simulink model "test03"  (R2022a)
settings: Solver=FixedStepAuto  StartTime=0.0  StopTime=0.05  FixedStep=1e-4  RelTol=1e-3
values from neighbouring files (symbols this model uses):
  A = 0.01    [parameter_load3.m]
  d = 2.6    [parameter_load3.m]
  I = 3055e-8    (= 3.055e-05)    [parameter_load3.m]
  jv = 1064400    [parameter_load3.m]
  kf = 6.67e7    (= 66700000)    [parameter_load3.m]
  kv = 400000    [parameter_load3.m]
  L = 210    [parameter_load3.m]
  m = 7800*(7684e-6)    (= 59.9352)    [parameter_load3.m]
  mv = 42400    [parameter_load3.m]
  position = 0.5*L    (= 105)    [parameter_load3.m]
  r = 840/1000/2    (= 0.42)    [parameter_load3.m]
  v = 100/3.6    (= 27.7777777778)    [parameter_load3.m]
(part 49 of 73)

== system test03/Subsystem8/Subsystem/Subsystem/Subsystem5/Subsystem2 ==
  An1 : Inport
  theta_n1 : Inport  Port=2
  theta_n2 : Inport  Port=3
  theta_n3 : Inport  Port=4
  Sn : Inport  Port=5
  static_n : Inport  Port=6
  Omiga_bn : Inport  Port=7
  Omiga_n : Inport  Port=8
  Add : Sum  Inputs=-+
  Add1 : Sum  Inputs=+-+-
  Add{2..4,6} (4 alike) : Sum
  Add5 : Sum  Inputs=+-
  Clock : Clock
  Clock1 : Clock
  Clock2 : Clock
  Constant : Constant
  Cos : Trigonometry  Operator=cos
  Cos1 : Trigonometry  Operator=cos
  Cos2 : Trigonometry  Operator=cos
  Divide : Product  Inputs=/*
  Gain : Gain  Gain=2
  Gain1 : Gain  Gain=2
  Product{,1..5} (6 alike) : Product
  Square : Math  Operator=square
  Out1 : Outport
  wires:
  Divide/1 -> Product5/1
  Add1/1 -> Product5/2
  Product5/1 -> Out1/1
  Product4/1 -> Add1/2
  Cos2/1 -> Product4/1
  Add6/1 -> Cos2/1
  Product3/1 -> Add6/1
  Clock2/1 -> Product3/2
  Add5/1 -> Product3/1
  theta_n2/1 -> Add6/2
  Product2/1 -> Add1/3
  Cos1/1 -> Product2/2
  Add4/1 -> Cos1/1
  theta_n3/1 -> Add4/2
  Product1/1 -> Add4/1
  Add3/1 -> Product1/1
  Clock1/1 -> Product1/2
  Omiga_bn/1 -> Add3/1, Add5/1
  Cos/1 -> Add1/4
  Add2/1 -> Cos/1
  theta_n1/1 -> Add2/2
  Product/1 -> Add2/1
  Clock/1 -> Product/2
  Gain1/1 -> Product/1
  Omiga_n/1 -> Gain1/1, Add3/2, Add5/2
  An1/1 -> Add1/1
  Gain/1 -> Divide/1
  Add/1 -> Gain/1
  Constant/1 -> Add/2
  Square/1 -> Add/1
  Sn/1 -> Product2/1, Product4/2, Square/1
  static_n/1 -> Divide/2

== system test03/Subsystem8/Subsystem/Subsystem/Subsystem5/Subsystem3 ==
  An1 : Inport
  theta_n1 : Inport  Port=2
  theta_n2 : Inport  Port=3
  theta_n3 : Inport  Port=4
  Sn : Inport  Port=5
  static_n : Inport  Port=6
  Omiga_bn : Inport  Port=7
  Omiga_n : Inport  Port=8
  Add : Sum  Inputs=-+
  Add1 : Sum  Inputs=+-+-
  Add{2..4,6} (4 alike) : Sum
  Add5 : Sum  Inputs=+-
  Clock : Clock
  Clock1 : Clock
  Clock2 : Clock
  Constant : Constant
  Cos : Trigonometry  Operator=cos
  Cos1 : Trigonometry  Operator=cos
  Cos2 : Trigonometry  Operator=cos
  Divide : Product  Inputs=/*
  Gain : Gain  Gain=2
  Gain1 : Gain  Gain=2
  Product{,1..5} (6 alike) : Product
  Square : Math  Operator=square
  Out1 : Outport
  wires:
  static_n/1 -> Divide/2
  Sn/1 -> Square/1, Product4/2, Product2/1
  Square/1 -> Add/1
  Constant/1 -> Add/2
  Add/1 -> Gain/1
  Gain/1 -> Divide/1
  An1/1 -> Add1/1
  Omiga_n/1 -> Add5/2, Add3/2, Gain1/1
  Gain1/1 -> Product/1
  Clock/1 -> Product/2
  Product/1 -> Add2/1
  theta_n1/1 -> Add2/2
  Add2/1 -> Cos/1
  Cos/1 -> Add1/4
  Omiga_bn/1 -> Add5/1, Add3/1
  Clock1/1 -> Product1/2
  Add3/1 -> Product1/1
  Product1/1 -> Add4/1
  theta_n3/1 -> Add4/2
  Add4/1 -> Cos1/1
  Cos1/1 -> Product2/2
  Product2/1 -> Add1/3
  theta_n2/1 -> Add6/2
  Add5/1 -> Product3/1
  Clock2/1 -> Product3/2
  Product3/1 -> Add6/1
  Add6/1 -> Cos2/1
  Cos2/1 -> Product4/1
  Product4/1 -> Add1/2
  Product5/1 -> Out1/1
  Add1/1 -> Product5/2
  Divide/1 -> Product5/1

== system test03/Subsystem8/Subsystem/Subsystem/Subsystem6/Subsystem ==
  imod : Inport
  r : Inport  Port=2
  k : Inport  Port=3
  F : Inport  Port=4
  Add : Sum  Inputs=+-
  Add1 : Sum
  Add2 : Sum
  Add3 : Sum  Inputs=-+
  Add4 : Sum  Inputs=-+
  Add5 : Sum  Inputs=--
  Constant : Constant
  Constant1 : Constant  Value=0
  Constant2 : Constant  Value=4
  Constant3 : Constant  Value=kf/m
  Cos : Trigonometry  Operator=cos
  Divide : Product  Inputs=*/
  Divide1 : Product  Inputs=*/
  Gain : Gain  Gain=d
  Gain1 : Gain  Gain=pi/L
  Gain2 : Gain  Gain=E*I/m
  Gain3 : Gain  Gain=v*pi/L
  Gain4 : Gain  Gain=-2/(m*L)
  Gain5 : Gain  Gain=-1
  Gain6 : Gain  Gain=1/v
  Power : Math  Operator=pow
  Product{1..6} (6 alike) : Product
  Sqrt : Sqrt
  Square1 : Math  Operator=square
  Switch : Switch  Criteria=u2 > Threshold  Threshold=1  SaturateOnIntegerOverflow=off  InputSameDT=off
  An1 : Outport
  theta_n1 : Outport  Port=2
  theta_n2 : Outport  Port=3
  theta_n3 : Outport  Port=4
  Sn : Outport  Port=5
  static_n : Outport  Port=6
  Omiga_bn : Outport  Port=7
  Omiga_n : Outport  Port=8
  wires:
  k/1 -> Add/1
  Constant/1 -> Add/2
  Add/1 -> Gain/1
  Gain/1 -> Gain6/1
  r/1 -> Switch/2
  Constant1/1 -> Switch/3
  [tk] -> Switch/1
  Switch/1 -> [tr]
  Product4/1 -> Cos/1
  [tr] -> Add4/1
  Sqrt/1 -> Omiga_bn/1, [Omiga_bn]
  Constant2/1 -> Power/2
  Gain2/1 -> Add1/1
  Add4/1 -> Product4/2
  Add1/1 -> Sqrt/1
  Gain4/1 -> Divide1/1
  F/1 -> Gain4/1
  [imod] -> Gain1/1
  Divide/1 -> Sn/1
  Constant3/1 -> Add1/2
  Divide1/1 -> static_n/1
  Gain1/1 -> Power/1
  Gain3/1 -> Omiga_n/1, [Omiga_n]
  Power/1 -> Gain2/1
  [Omiga_bn] -> Square1/1
  Square1/1 -> Divide1/2
  [tk] -> Add4/2
  [Omiga_n] -> Divide/1
  [Omiga_n] -> Product4/1
  [Omiga_bn] -> Divide/2
  [imod] -> Gain3/1
  imod/1 -> [imod]
  Cos/1 -> An1/1
  [Omiga_n] -> Product1/1
  [tk] -> Add2/2
  Add2/1 -> Product1/2
  [tr] -> Add2/1
  Product1/1 -> Gain5/1
  Gain5/1 -> theta_n1/1
  [Omiga_bn] -> Product2/1
  [tk] -> Product2/2
  Product2/1 -> Add3/1
  Add3/1 -> theta_n2/1
  Product3/1 -> Add3/2
  [tr] -> Product3/2
  [Omiga_n] -> Product3/1
  [Omiga_n] -> Product6/1
  [tr] -> Product6/2
  Product6/1 -> Add5/2
  Add5/1 -> theta_n3/1
  Product5/1 -> Add5/1
  [tk] -> Product5/2
  [Omiga_bn] -> Product5/1
  Gain6/1 -> [tk]

== system test03/Subsystem8/Subsystem/Subsystem/Subsystem6/Subsystem1 ==
  imod : Inport
  r : Inport  Port=2
  k : Inport  Port=3
  F : Inport  Port=4
  Add : Sum  Inputs=+-
  Add1 : Sum
  Add2 : Sum
  Add3 : Sum  Inputs=-+
  Add4 : Sum  Inputs=-+
  Add5 : Sum  Inputs=--
  Constant : Constant
  Constant1 : Constant  Value=0
  Constant2 : Constant  Value=4
  Constant3 : Constant  Value=kf/m
  Cos : Trigonometry  Operator=cos
  Divide : Product  Inputs=*/
  Divide1 : Product  Inputs=*/
  Gain : Gain  Gain=d
  Gain1 : Gain  Gain=pi/L
  Gain2 : Gain  Gain=E*I/m
  Gain3 : Gain  Gain=v*pi/L
  Gain4 : Gain  Gain=-2/(m*L)
  Gain5 : Gain  Gain=-1
  Gain6 : Gain  Gain=1/v
  Power : Math  Operator=pow
  Product{1..6} (6 alike) : Product
  Sqrt : Sqrt
  Square1 : Math  Operator=square
  Switch : Switch  Criteria=u2 > Threshold  Threshold=1  SaturateOnIntegerOverflow=off  InputSameDT=off
  An1 : Outport
  theta_n1 : Outport  Port=2
  theta_n2 : Outport  Port=3
  theta_n3 : Outport  Port=4
  Sn : Outport  Port=5
  static_n : Outport  Port=6
  Omiga_bn : Outport  Port=7
  Omiga_n : Outport  Port=8
  wires:
  Gain6/1 -> [tk]
  [Omiga_bn] -> Product5/1
  [tk] -> Product5/2
  Product5/1 -> Add5/1
  Add5/1 -> theta_n3/1
  Product6/1 -> Add5/2
  [tr] -> Product6/2
  [Omiga_n] -> Product6/1
  [Omiga_n] -> Product3/1
  [tr] -> Product3/2
  Product3/1 -> Add3/2
  Add3/1 -> theta_n2/1
  Product2/1 -> Add3/1
  [tk] -> Product2/2
  [Omiga_bn] -> Product2/1
  Gain5/1 -> theta_n1/1
  Product1/1 -> Gain5/1
  [tr] -> Add2/1
  Add2/1 -> Product1/2
  [tk] -> Add2/2
  [Omiga_n] -> Product1/1
  Cos/1 -> An1/1
  imod/1 -> [imod]
  [imod] -> Gain3/1
  [Omiga_bn] -> Divide/2
  [Omiga_n] -> Product4/1
  [Omiga_n] -> Divide/1
  [tk] -> Add4/2
  Square1/1 -> Divide1/2
  [Omiga_bn] -> Square1/1
  Power/1 -> Gain2/1
  Gain3/1 -> [Omiga_n], Omiga_n/1
  Gain1/1 -> Power/1
  Divide1/1 -> static_n/1
  Constant3/1 -> Add1/2
  Divide/1 -> Sn/1
  [imod] -> Gain1/1
  F/1 -> Gain4/1
  Gain4/1 -> Divide1/1
  Add1/1 -> Sqrt/1
  Add4/1 -> Product4/2
  Gain2/1 -> Add1/1
  Constant2/1 -> Power/2
  Sqrt/1 -> [Omiga_bn], Omiga_bn/1
  [tr] -> Add4/1
  Product4/1 -> Cos/1
  Switch/1 -> [tr]
  [tk] -> Switch/1
  Constant1/1 -> Switch/3
  r/1 -> Switch/2
  Gain/1 -> Gain6/1
  Add/1 -> Gain/1
  Constant/1 -> Add/2
  k/1 -> Add/1

== system test03/Subsystem8/Subsystem/Subsystem/Subsystem6/Subsystem2 ==
  An1 : Inport
  theta_n1 : Inport  Port=2
  theta_n2 : Inport  Port=3
  theta_n3 : Inport  Port=4
  Sn : Inport  Port=5
  static_n : Inport  Port=6
  Omiga_bn : Inport  Port=7
  Omiga_n : Inport  Port=8
  Add : Sum  Inputs=-+
  Add1 : Sum  Inputs=+-+-
  Add{2..4,6} (4 alike) : Sum
  Add5 : Sum  Inputs=+-
  Clock : Clock
  Clock1 : Clock
  Clock2 : Clock
  Constant : Constant
  Cos : Trigonometry  Operator=cos
  Cos1 : Trigonometry  Operator=cos
  Cos2 : Trigonometry  Operator=cos
  Divide : Product  Inputs=/*
  Gain : Gain  Gain=2
  Gain1 : Gain  Gain=2
  Product{,1..5} (6 alike) : Product
  Square : Math  Operator=square
  Out1 : Outport
  wires:
  static_n/1 -> Divide/2
  Sn/1 -> Square/1, Product4/2, Product2/1
  Square/1 -> Add/1
  Constant/1 -> Add/2
  Add/1 -> Gain/1
  Gain/1 -> Divide/1
  An1/1 -> Add1/1
  Omiga_n/1 -> Add5/2, Add3/2, Gain1/1
  Gain1/1 -> Product/1
  Clock/1 -> Product/2
  Product/1 -> Add2/1
  theta_n1/1 -> Add2/2
  Add2/1 -> Cos/1
  Cos/1 -> Add1/4
  Omiga_bn/1 -> Add5/1, Add3/1
  Clock1/1 -> Product1/2
  Add3/1 -> Product1/1
  Product1/1 -> Add4/1
  theta_n3/1 -> Add4/2
  Add4/1 -> Cos1/1
  Cos1/1 -> Product2/2
  Product2/1 -> Add1/3
  theta_n2/1 -> Add6/2
  Add5/1 -> Product3/1
  Clock2/1 -> Product3/2
  Product3/1 -> Add6/1
  Add6/1 -> Cos2/1
  Cos2/1 -> Product4/1
  Product4/1 -> Add1/2
  Product5/1 -> Out1/1
  Add1/1 -> Product5/2
  Divide/1 -> Product5/1

== system test03/Subsystem8/Subsystem/Subsystem/Subsystem6/Subsystem3 ==
  An1 : Inport
  theta_n1 : Inport  Port=2
  theta_n2 : Inport  Port=3
  theta_n3 : Inport  Port=4
  Sn : Inport  Port=5
  static_n : Inport  Port=6
  Omiga_bn : Inport  Port=7
  Omiga_n : Inport  Port=8
  Add : Sum  Inputs=-+
  Add1 : Sum  Inputs=+-+-
  Add{2..4,6} (4 alike) : Sum
  Add5 : Sum  Inputs=+-
  Clock : Clock
  Clock1 : Clock
  Clock2 : Clock
  Constant : Constant
  Cos : Trigonometry  Operator=cos
  Cos1 : Trigonometry  Operator=cos
  Cos2 : Trigonometry  Operator=cos
  Divide : Product  Inputs=/*
  Gain : Gain  Gain=2
  Gain1 : Gain  Gain=2
  Product{,1..5} (6 alike) : Product
  Square : Math  Operator=square
  Out1 : Outport
  wires:
  Divide/1 -> Product5/1
  Add1/1 -> Product5/2
  Product5/1 -> Out1/1
  Product4/1 -> Add1/2
  Cos2/1 -> Product4/1
  Add6/1 -> Cos2/1
  Product3/1 -> Add6/1
  Clock2/1 -> Product3/2
  Add5/1 -> Product3/1
  theta_n2/1 -> Add6/2
  Product2/1 -> Add1/3
  Cos1/1 -> Product2/2
  Add4/1 -> Cos1/1
  theta_n3/1 -> Add4/2
  Product1/1 -> Add4/1
  Add3/1 -> Product1/1
  Clock1/1 -> Product1/2
  Omiga_bn/1 -> Add3/1, Add5/1
  Cos/1 -> Add1/4
  Add2/1 -> Cos/1
  theta_n1/1 -> Add2/2
  Product/1 -> Add2/1
  Clock/1 -> Product/2
  Gain1/1 -> Product/1
  Omiga_n/1 -> Gain1/1, Add3/2, Add5/2
  An1/1 -> Add1/1
  Gain/1 -> Divide/1
  Add/1 -> Gain/1
  Constant/1 -> Add/2
  Square/1 -> Add/1
  Sn/1 -> Product2/1, Product4/2, Square/1
  static_n/1 -> Divide/2

== system test03/Subsystem8/Subsystem/Subsystem/Subsystem7/Subsystem ==
  imod : Inport
  r : Inport  Port=2
  k : Inport  Port=3
  F : Inport  Port=4
  Add : Sum  Inputs=+-
  Add1 : Sum
  Add2 : Sum
  Add3 : Sum  Inputs=-+
  Add4 : Sum  Inputs=-+
  Add5 : Sum  Inputs=--
  Constant : Constant
  Constant1 : Constant  Value=0
  Constant2 : Constant  Value=4
  Constant3 : Constant  Value=kf/m
  Cos : Trigonometry  Operator=cos
  Divide : Product  Inputs=*/
  Divide1 : Product  Inputs=*/
  Gain : Gain  Gain=d
  Gain1 : Gain  Gain=pi/L
  Gain2 : Gain  Gain=E*I/m
  Gain3 : Gain  Gain=v*pi/L
  Gain4 : Gain  Gain=-2/(m*L)
  Gain5 : Gain  Gain=-1
  Gain6 : Gain  Gain=1/v
  Power : Math  Operator=pow
  Product{1..6} (6 alike) : Product
  Sqrt : Sqrt
  Square1 : Math  Operator=square
  Switch : Switch  Criteria=u2 > Threshold  Threshold=1  SaturateOnIntegerOverflow=off  InputSameDT=off
  An1 : Outport
  theta_n1 : Outport  Port=2
  theta_n2 : Outport  Port=3
  theta_n3 : Outport  Port=4
  Sn : Outport  Port=5
  static_n : Outport  Port=6
  Omiga_bn : Outport  Port=7
  Omiga_n : Outport  Port=8
  wires:
  k/1 -> Add/1
  Constant/1 -> Add/2
  Add/1 -> Gain/1
  Gain/1 -> Gain6/1
  r/1 -> Switch/2
  Constant1/1 -> Switch/3
  [tk] -> Switch/1
  Switch/1 -> [tr]
  Product4/1 -> Cos/1
  [tr] -> Add4/1
  Sqrt/1 -> Omiga_bn/1, [Omiga_bn]
  Constant2/1 -> Power/2
  Gain2/1 -> Add1/1
  Add4/1 -> Product4/2
  Add1/1 -> Sqrt/1
  Gain4/1 -> Divide1/1
  F/1 -> Gain4/1
  [imod] -> Gain1/1
  Divide/1 -> Sn/1
  Constant3/1 -> Add1/2
  Divide1/1 -> static_n/1
  Gain1/1 -> Power/1
  Gain3/1 -> Omiga_n/1, [Omiga_n]
  Power/1 -> Gain2/1
  [Omiga_bn] -> Square1/1
  Square1/1 -> Divide1/2
  [tk] -> Add4/2
  [Omiga_n] -> Divide/1
  [Omiga_n] -> Product4/1
  [Omiga_bn] -> Divide/2
  [imod] -> Gain3/1
  imod/1 -> [imod]
  Cos/1 -> An1/1
  [Omiga_n] -> Product1/1
  [tk] -> Add2/2
  Add2/1 -> Product1/2
  [tr] -> Add2/1
  Product1/1 -> Gain5/1
  Gain5/1 -> theta_n1/1
  [Omiga_bn] -> Product2/1
  [tk] -> Product2/2
  Product2/1 -> Add3/1
  Add3/1 -> theta_n2/1
  Product3/1 -> Add3/2
  [tr] -> Product3/2
  [Omiga_n] -> Product3/1
  [Omiga_n] -> Product6/1
  [tr] -> Product6/2
  Product6/1 -> Add5/2
  Add5/1 -> theta_n3/1
  Product5/1 -> Add5/1
  [tk] -> Product5/2
  [Omiga_bn] -> Product5/1
  Gain6/1 -> [tk]

== system test03/Subsystem8/Subsystem/Subsystem/Subsystem7/Subsystem1 ==
  imod : Inport
  r : Inport  Port=2
  k : Inport  Port=3
  F : Inport  Port=4
  Add : Sum  Inputs=+-
  Add1 : Sum
  Add2 : Sum
  Add3 : Sum  Inputs=-+
  Add4 : Sum  Inputs=-+
  Add5 : Sum  Inputs=--
  Constant : Constant
  Constant1 : Constant  Value=0
  Constant2 : Constant  Value=4
  Constant3 : Constant  Value=kf/m
  Cos : Trigonometry  Operator=cos
  Divide : Product  Inputs=*/
  Divide1 : Product  Inputs=*/
  Gain : Gain  Gain=d
  Gain1 : Gain  Gain=pi/L
  Gain2 : Gain  Gain=E*I/m
  Gain3 : Gain  Gain=v*pi/L
  Gain4 : Gain  Gain=-2/(m*L)
  Gain5 : Gain  Gain=-1
  Gain6 : Gain  Gain=1/v
  Power : Math  Operator=pow
  Product{1..6} (6 alike) : Product
  Sqrt : Sqrt
  Square1 : Math  Operator=square
  Switch : Switch  Criteria=u2 > Threshold  Threshold=1  SaturateOnIntegerOverflow=off  InputSameDT=off
  An1 : Outport
  theta_n1 : Outport  Port=2
  theta_n2 : Outport  Port=3
  theta_n3 : Outport  Port=4
  Sn : Outport  Port=5
  static_n : Outport  Port=6
  Omiga_bn : Outport  Port=7
  Omiga_n : Outport  Port=8
  wires:
  Gain6/1 -> [tk]
  [Omiga_bn] -> Product5/1
  [tk] -> Product5/2
  Product5/1 -> Add5/1
  Add5/1 -> theta_n3/1
  Product6/1 -> Add5/2
  [tr] -> Product6/2
  [Omiga_n] -> Product6/1
  [Omiga_n] -> Product3/1
  [tr] -> Product3/2
  Product3/1 -> Add3/2
  Add3/1 -> theta_n2/1
  Product2/1 -> Add3/1
  [tk] -> Product2/2
  [Omiga_bn] -> Product2/1
  Gain5/1 -> theta_n1/1
  Product1/1 -> Gain5/1
  [tr] -> Add2/1
  Add2/1 -> Product1/2
  [tk] -> Add2/2
  [Omiga_n] -> Product1/1
  Cos/1 -> An1/1
  imod/1 -> [imod]
  [imod] -> Gain3/1
  [Omiga_bn] -> Divide/2
  [Omiga_n] -> Product4/1
  [Omiga_n] -> Divide/1
  [tk] -> Add4/2
  Square1/1 -> Divide1/2
  [Omiga_bn] -> Square1/1
  Power/1 -> Gain2/1
  Gain3/1 -> [Omiga_n], Omiga_n/1
  Gain1/1 -> Power/1
  Divide1/1 -> static_n/1
  Constant3/1 -> Add1/2
  Divide/1 -> Sn/1
  [imod] -> Gain1/1
  F/1 -> Gain4/1
  Gain4/1 -> Divide1/1
  Add1/1 -> Sqrt/1
  Add4/1 -> Product4/2
  Gain2/1 -> Add1/1
  Constant2/1 -> Power/2
  Sqrt/1 -> [Omiga_bn], Omiga_bn/1
  [tr] -> Add4/1
  Product4/1 -> Cos/1
  Switch/1 -> [tr]
  [tk] -> Switch/1
  Constant1/1 -> Switch/3
  r/1 -> Switch/2
  Gain/1 -> Gain6/1
  Add/1 -> Gain/1
  Constant/1 -> Add/2
  k/1 -> Add/1

== system test03/Subsystem8/Subsystem/Subsystem/Subsystem7/Subsystem2 ==
  An1 : Inport
  theta_n1 : Inport  Port=2
  theta_n2 : Inport  Port=3
  theta_n3 : Inport  Port=4
  Sn : Inport  Port=5
  static_n : Inport  Port=6
  Omiga_bn : Inport  Port=7
  Omiga_n : Inport  Port=8
  Add : Sum  Inputs=-+
  Add1 : Sum  Inputs=+-+-
  Add{2..4,6} (4 alike) : Sum
  Add5 : Sum  Inputs=+-
  Clock : Clock
  Clock1 : Clock
  Clock2 : Clock
  Constant : Constant
  Cos : Trigonometry  Operator=cos
  Cos1 : Trigonometry  Operator=cos
  Cos2 : Trigonometry  Operator=cos
  Divide : Product  Inputs=/*
  Gain : Gain  Gain=2
  Gain1 : Gain  Gain=2
  Product{,1..5} (6 alike) : Product
  Square : Math  Operator=square
  Out1 : Outport
  wires:
  static_n/1 -> Divide/2
  Sn/1 -> Square/1, Product4/2, Product2/1
  Square/1 -> Add/1
  Constant/1 -> Add/2
  Add/1 -> Gain/1
  Gain/1 -> Divide/1
  An1/1 -> Add1/1
  Omiga_n/1 -> Add5/2, Add3/2, Gain1/1
  Gain1/1 -> Product/1
  Clock/1 -> Product/2
  Product/1 -> Add2/1
  theta_n1/1 -> Add2/2
  Add2/1 -> Cos/1
  Cos/1 -> Add1/4
  Omiga_bn/1 -> Add5/1, Add3/1
  Clock1/1 -> Product1/2
  Add3/1 -> Product1/1
  Product1/1 -> Add4/1
  theta_n3/1 -> Add4/2
  Add4/1 -> Cos1/1
  Cos1/1 -> Product2/2
  Product2/1 -> Add1/3
  theta_n2/1 -> Add6/2
  Add5/1 -> Product3/1
  Clock2/1 -> Product3/2
  Product3/1 -> Add6/1
  Add6/1 -> Cos2/1
  Cos2/1 -> Product4/1
  Product4/1 -> Add1/2
  Product5/1 -> Out1/1
  Add1/1 -> Product5/2
  Divide/1 -> Product5/1

== system test03/Subsystem8/Subsystem/Subsystem/Subsystem7/Subsystem3 ==
  An1 : Inport
  theta_n1 : Inport  Port=2
  theta_n2 : Inport  Port=3
  theta_n3 : Inport  Port=4
  Sn : Inport  Port=5
  static_n : Inport  Port=6
  Omiga_bn : Inport  Port=7
  Omiga_n : Inport  Port=8
  Add : Sum  Inputs=-+
  Add1 : Sum  Inputs=+-+-
  Add{2..4,6} (4 alike) : Sum
  Add5 : Sum  Inputs=+-
  Clock : Clock
  Clock1 : Clock
  Clock2 : Clock
  Constant : Constant
  Cos : Trigonometry  Operator=cos
  Cos1 : Trigonometry  Operator=cos
  Cos2 : Trigonometry  Operator=cos
  Divide : Product  Inputs=/*
  Gain : Gain  Gain=2
  Gain1 : Gain  Gain=2
  Product{,1..5} (6 alike) : Product
  Square : Math  Operator=square
  Out1 : Outport
  wires:
  Divide/1 -> Product5/1
  Add1/1 -> Product5/2
  Product5/1 -> Out1/1
  Product4/1 -> Add1/2
  Cos2/1 -> Product4/1
  Add6/1 -> Cos2/1
  Product3/1 -> Add6/1
  Clock2/1 -> Product3/2
  Add5/1 -> Product3/1
  theta_n2/1 -> Add6/2
  Product2/1 -> Add1/3
  Cos1/1 -> Product2/2
  Add4/1 -> Cos1/1
  theta_n3/1 -> Add4/2
  Product1/1 -> Add4/1
  Add3/1 -> Product1/1
  Clock1/1 -> Product1/2
  Omiga_bn/1 -> Add3/1, Add5/1
  Cos/1 -> Add1/4
  Add2/1 -> Cos/1
  theta_n1/1 -> Add2/2
  Product/1 -> Add2/1
  Clock/1 -> Product/2
  Gain1/1 -> Product/1
  Omiga_n/1 -> Gain1/1, Add3/2, Add5/2
  An1/1 -> Add1/1
  Gain/1 -> Divide/1
  Add/1 -> Gain/1
  Constant/1 -> Add/2
  Square/1 -> Add/1
  Sn/1 -> Product2/1, Product4/2, Square/1
  static_n/1 -> Divide/2

== system test03/Subsystem8/Subsystem/Subsystem/Subsystem8/Subsystem ==
  imod : Inport
  r : Inport  Port=2
  k : Inport  Port=3
  F : Inport  Port=4
  Add : Sum  Inputs=+-
  Add1 : Sum
  Add2 : Sum
  Add3 : Sum  Inputs=-+
  Add4 : Sum  Inputs=-+
  Add5 : Sum  Inputs=--
  Constant : Constant
  Constant1 : Constant  Value=0
  Constant2 : Constant  Value=4
  Constant3 : Constant  Value=kf/m
  Cos : Trigonometry  Operator=cos
  Divide : Product  Inputs=*/
  Divide1 : Product  Inputs=*/
  Gain : Gain  Gain=d
  Gain1 : Gain  Gain=pi/L
  Gain2 : Gain  Gain=E*I/m
  Gain3 : Gain  Gain=v*pi/L
  Gain4 : Gain  Gain=-2/(m*L)
  Gain5 : Gain  Gain=-1
  Gain6 : Gain  Gain=1/v
  Power : Math  Operator=pow
  Product{1..6} (6 alike) : Product
  Sqrt : Sqrt
  Square1 : Math  Operator=square
  Switch : Switch  Criteria=u2 > Threshold  Threshold=1  SaturateOnIntegerOverflow=off  InputSameDT=off
  An1 : Outport
  theta_n1 : Outport  Port=2
  theta_n2 : Outport  Port=3
  theta_n3 : Outport  Port=4
  Sn : Outport  Port=5
  static_n : Outport  Port=6
  Omiga_bn : Outport  Port=7
  Omiga_n : Outport  Port=8
  wires:
  Gain6/1 -> [tk]
  [Omiga_bn] -> Product5/1
  [tk] -> Product5/2
  Product5/1 -> Add5/1
  Add5/1 -> theta_n3/1
  Product6/1 -> Add5/2
  [tr] -> Product6/2
  [Omiga_n] -> Product6/1
  [Omiga_n] -> Product3/1
  [tr] -> Product3/2
  Product3/1 -> Add3/2
  Add3/1 -> theta_n2/1
  Product2/1 -> Add3/1
  [tk] -> Product2/2
  [Omiga_bn] -> Product2/1
  Gain5/1 -> theta_n1/1
  Product1/1 -> Gain5/1
  [tr] -> Add2/1
  Add2/1 -> Product1/2
  [tk] -> Add2/2
  [Omiga_n] -> Product1/1
  Cos/1 -> An1/1
  imod/1 -> [imod]
  [imod] -> Gain3/1
  [Omiga_bn] -> Divide/2
  [Omiga_n] -> Product4/1
  [Omiga_n] -> Divide/1
  [tk] -> Add4/2
  Square1/1 -> Divide1/2
  [Omiga_bn] -> Square1/1
  Power/1 -> Gain2/1
  Gain3/1 -> [Omiga_n], Omiga_n/1
  Gain1/1 -> Power/1
  Divide1/1 -> static_n/1
  Constant3/1 -> Add1/2
  Divide/1 -> Sn/1
  [imod] -> Gain1/1
  F/1 -> Gain4/1
  Gain4/1 -> Divide1/1
  Add1/1 -> Sqrt/1
  Add4/1 -> Product4/2
  Gain2/1 -> Add1/1
  Constant2/1 -> Power/2
  Sqrt/1 -> [Omiga_bn], Omiga_bn/1
  [tr] -> Add4/1
  Product4/1 -> Cos/1
  Switch/1 -> [tr]
  [tk] -> Switch/1
  Constant1/1 -> Switch/3
  r/1 -> Switch/2
  Gain/1 -> Gain6/1
  Add/1 -> Gain/1
  Constant/1 -> Add/2
  k/1 -> Add/1

== system test03/Subsystem8/Subsystem/Subsystem/Subsystem8/Subsystem1 ==
  imod : Inport
  r : Inport  Port=2
  k : Inport  Port=3
  F : Inport  Port=4
  Add : Sum  Inputs=+-
  Add1 : Sum
  Add2 : Sum
  Add3 : Sum  Inputs=-+
  Add4 : Sum  Inputs=-+
  Add5 : Sum  Inputs=--
  Constant : Constant
  Constant1 : Constant  Value=0
  Constant2 : Constant  Value=4
  Constant3 : Constant  Value=kf/m
  Cos : Trigonometry  Operator=cos
  Divide : Product  Inputs=*/
  Divide1 : Product  Inputs=*/
  Gain : Gain  Gain=d
  Gain1 : Gain  Gain=pi/L
  Gain2 : Gain  Gain=E*I/m
  Gain3 : Gain  Gain=v*pi/L
  Gain4 : Gain  Gain=-2/(m*L)
  Gain5 : Gain  Gain=-1
  Gain6 : Gain  Gain=1/v
  Power : Math  Operator=pow
  Product{1..6} (6 alike) : Product
  Sqrt : Sqrt
  Square1 : Math  Operator=square
  Switch : Switch  Criteria=u2 > Threshold  Threshold=1  SaturateOnIntegerOverflow=off  InputSameDT=off
  An1 : Outport
  theta_n1 : Outport  Port=2
  theta_n2 : Outport  Port=3
  theta_n3 : Outport  Port=4
  Sn : Outport  Port=5
  static_n : Outport  Port=6
  Omiga_bn : Outport  Port=7
  Omiga_n : Outport  Port=8
  wires:
  k/1 -> Add/1
  Constant/1 -> Add/2
  Add/1 -> Gain/1
  Gain/1 -> Gain6/1
  r/1 -> Switch/2
  Constant1/1 -> Switch/3
  [tk] -> Switch/1
  Switch/1 -> [tr]
  Product4/1 -> Cos/1
  [tr] -> Add4/1
  Sqrt/1 -> Omiga_bn/1, [Omiga_bn]
  Constant2/1 -> Power/2
  Gain2/1 -> Add1/1
  Add4/1 -> Product4/2
  Add1/1 -> Sqrt/1
  Gain4/1 -> Divide1/1
  F/1 -> Gain4/1
  [imod] -> Gain1/1
  Divide/1 -> Sn/1
  Constant3/1 -> Add1/2
  Divide1/1 -> static_n/1
  Gain1/1 -> Power/1
  Gain3/1 -> Omiga_n/1, [Omiga_n]
  Power/1 -> Gain2/1
  [Omiga_bn] -> Square1/1
  Square1/1 -> Divide1/2
  [tk] -> Add4/2
  [Omiga_n] -> Divide/1
  [Omiga_n] -> Product4/1
  [Omiga_bn] -> Divide/2
  [imod] -> Gain3/1
  imod/1 -> [imod]
  Cos/1 -> An1/1
  [Omiga_n] -> Product1/1
  [tk] -> Add2/2
  Add2/1 -> Product1/2
  [tr] -> Add2/1
  Product1/1 -> Gain5/1
  Gain5/1 -> theta_n1/1
  [Omiga_bn] -> Product2/1
  [tk] -> Product2/2
  Product2/1 -> Add3/1
  Add3/1 -> theta_n2/1
  Product3/1 -> Add3/2
  [tr] -> Product3/2
  [Omiga_n] -> Product3/1
  [Omiga_n] -> Product6/1
  [tr] -> Product6/2
  Product6/1 -> Add5/2
  Add5/1 -> theta_n3/1
  Product5/1 -> Add5/1
  [tk] -> Product5/2
  [Omiga_bn] -> Product5/1
  Gain6/1 -> [tk]
